simulink model "ofdm_txrx_supermimo_coded"  (Simulink 7.7)
settings: Solver=VariableStepDiscrete  SolverMode=SingleTasking  StartTime=0.0  StopTime=2*simOnly_simLength  RelTol=1e-3
PostLoadFcn:
  | ofdm_rx_supermimo_init
InitFcn:
  | ofdm_rx_supermimo_init
StartFcn:
  | ofdm_rx_supermimo_init
values from neighbouring files (symbols this model uses):
  ActionID_AFTransmit = 31    [ofdm_tx_supermimo_init.m]
  ActionID_Disabled = 0    [ofdm_tx_supermimo_init.m]
  ActionID_SetFlagA = 62    [ofdm_tx_supermimo_init.m]
  ActionID_SetFlagB = 61    [ofdm_tx_supermimo_init.m]
  BER_RAM_init_size = 2048    [ofdm_tx_supermimo_init.m]
  channel_h = [1 0; 1 0] * 0.3    [ofdm_tx_supermimo_init.m]
  CPLength = 16    [ofdm_tx_supermimo_init.m]
  longCorr_coef_i = [-4*real(LTS) 0]    [ofdm_rx_supermimo_init.m]
  longCorr_coef_q = [4*imag(LTS) 0]    [ofdm_rx_supermimo_init.m]
  max_CP_length = 16    [ofdm_tx_supermimo_init.m]
  max_num_baseRateSymbols = 31    [ofdm_tx_supermimo_init.m]
  max_num_subcarriers = 64    [ofdm_tx_supermimo_init.m]
  max_num_trainingSymbols = 15    [ofdm_tx_supermimo_init.m]
  max_numBytes = 16384    [ofdm_tx_supermimo_init.m]
  max_OFDM_symbols = 1023    [ofdm_tx_supermimo_init.m]
  modConstellation_qam16 = .75*[1 3 -1 -3]./3    [ofdm_tx_supermimo_init.m]
  modConstellation_qam64 = 0.875*[3 1 5 7 -3 -1 -5 -7]./7    [ofdm_tx_supermimo_init.m]
  modConstellation_qpsk = [1 -1]./sqrt(2)    [ofdm_tx_supermimo_init.m]
  numBaseRateSymbols = numBaseRateSymbols *2    [ofdm_tx_supermimo_init.m]
  numSubcarriers = 64    [ofdm_tx_supermimo_init.m]
  numTrainingSymbols = 2    [ofdm_tx_supermimo_init.m]
  pktTiming_count_payloadStart = pktTiming_count_lsStart + pktTiming_ls_num*pktTiming_ls_length    [ofdm_rx_supermimo_init.m]
  preamble = preamble_scale*[shortsyms_10 longsyms_2]    [ofdm_tx_supermimo_init.m]
  preamble_ROM = preamble_I_ROM + preamble_Q_ROM*2^16    [ofdm_tx_supermimo_init.m]
  RAM_init_size = max_numBytes    (= 16384)    [ofdm_tx_supermimo_init.m]
  RxDataScrambling_Seq = [40 198 78 63 82 173 102 245 48 111 172 115 147 230 216 93 72 65 62 2 205 242 122 90 128 83 105 97 73 10 5 252]    [ofdm_tx_supermimo_init.m]
  shortSymbol_time = shortSymbol_time(1:16).'    [ofdm_tx_supermimo_init.m]
  simOnly_numSamples = length(preamble)+( (numSubcarriers+CPLength)*(numTrainingSymbols + numBaseRateSymbols + numFullRateSymbols/2) )    [ofdm_tx_supermimo_init.m]
  train = [train train]    [ofdm_tx_supermimo_init.m]
  TxDataScrambling_Seq = [40 198 78 63 82 173 102 245 48 111 172 115 147 230 216 93 72 65 62 2 205 242 122 90 128 83 105 97 73 10 5 252]    [ofdm_tx_supermimo_init.m]
undefined symbols (not defined in the file or in its folder): AntA, AntB, BaseRate, Down, gcbh, numBuffs, numBytes, Up, xlBlockLoadCallback, xlGetNormalizedPeriod, xlipgetpartsetting
libraries: xbsIndex_r4 x5430
(part 30 of 49)

== system ofdm_txrx_supermimo_coded/OFDM Rx MIMO/FFT & Chan Est/Channel Estimation & Pilot Processing/H_B-B Estimate Buffer/Q Estimate Buffer ==
  note: data
  Est_Data : Inport
  Est_Addr : Inport  Port=2
  Est_WE : Inport  Port=3
  Rst : Inport  Port=4
  AddSub2 : xbsIndex_r4/AddSub  mode=Addition  use_carryin=off  use_carryout=off  en=off  latency=0  precision=User Defined  arith_type="Signed  (2's comp)"  n_bits=18  bin_pt=15  quantization=Truncate  overflow=Flag as error  dbl_ovrd=off  use_behavioral_HDL=on  hw_selection=Fabric  pipelined=off  use_rpm=on  block_type=addsub
      settings: xl_area=[0, 0, 0, 0, 0, 0, 0]  block_version=VER_STRING_GOES_HERE
  Constant Read State : xbsIndex_r4/Constant  const=0  gui_display_data_type=Fixed-point  arith_type=Boolean  n_bits=1  bin_pt=0  preci_type=Single  exp_width=8  frac_width=24  explicit_period=off  period=1  equ=P=C  opselect=C  inp2=PCIN>>17  opr=+  inp1=P  carry=CIN  dbl_ovrd=off  block_type=constant
      settings: block_version=VER_STRING_GOES_HERE
  Constant4 : xbsIndex_r4/Constant  const=0  gui_display_data_type=Fixed-point  arith_type=Signed (2's comp)  n_bits=18  bin_pt=15  preci_type=Single  exp_width=8  frac_width=24  explicit_period=off  period=1  equ=P=C  opselect=C  inp2=PCIN>>17  opr=+  inp1=P  carry=CIN  dbl_ovrd=off  block_type=constant
      settings: block_version=VER_STRING_GOES_HERE
  Convert1 : xbsIndex_r4/Convert  gui_display_data_type=Fixed-point  arith_type="Signed  (2's comp)"  n_bits=18  bin_pt=15  float_type=Single  exp_bits=8  fraction_bits=24  quantization=Truncate  overflow=Flag as error  en=off  latency=0  dbl_ovrd=off  pipeline=off  block_type=convert
      settings: xl_area=[0, 0, 0, 0, 0, 0, 0]  block_version=VER_STRING_GOES_HERE
  Convert2 : xbsIndex_r4/Convert  gui_display_data_type=Fixed-point  arith_type="Signed  (2's comp)"  n_bits=18  bin_pt=16  float_type=Single  exp_bits=8  fraction_bits=24  quantization=Truncate  overflow=Flag as error  en=off  latency=0  dbl_ovrd=off  pipeline=off  block_type=convert
      settings: xl_area=[0, 0, 0, 0, 0, 0, 0]  block_version=VER_STRING_GOES_HERE
  Delay3 : xbsIndex_r4/Delay  rst=off  infoeditControl=Selection of Reset will increase slice count due to use of real FFs and instead of SRLs  en=off  latency=1  dbl_ovrd=off  reg_retiming=off  block_type=delay
      settings: infoedit=Hardware notes: A delay line is a chain, each link of which is an SRL16 followed by a flip-flop. If register retiming is enabled, the delay line is a chain of flip-flops.  xl_area=[0, 0, 0, 0, 0, 0, 0]  block_version=VER_STRING_GOES_HERE
  Delay6 : xbsIndex_r4/Delay  rst=off  infoeditControl=Selection of Reset will increase slice count due to use of real FFs and instead of SRLs  en=off  latency=1  dbl_ovrd=off  reg_retiming=off  block_type=delay
      settings: infoedit=Hardware notes: A delay line is a chain, each link of which is an SRL16 followed by a flip-flop. If register retiming is enabled, the delay line is a chain of flip-flops.  xl_area=[0, 0, 0, 0, 0, 0, 0]  block_version=VER_STRING_GOES_HERE
  Delay7 : xbsIndex_r4/Delay  rst=off  infoeditControl=Selection of Reset will increase slice count due to use of real FFs and instead of SRLs  en=off  latency=1  dbl_ovrd=off  reg_retiming=off  block_type=delay
      settings: infoedit=Hardware notes: A delay line is a chain, each link of which is an SRL16 followed by a flip-flop. If register retiming is enabled, the delay line is a chain of flip-flops.  xl_area=[0, 0, 0, 0, 0, 0, 0]  block_version=VER_STRING_GOES_HERE
  Dual Port RAM : xbsIndex_r4/Dual Port RAM  depth=numSubcarriers  initVector=zeros(1,numSubcarriers)  distributed_mem=Block RAM  init_a=0  init_b=0  rst_a=off  rst_b=on  en_a=off  en_b=off  latency=1  write_mode_A=Read Before Write  write_mode_B=Read After Write  dbl_ovrd=off  optimize=Area  use_rpm=off  block_type=dpram
      settings: xl_area=[0, 0, 0, 0, 0, 0, 0]  block_version=VER_STRING_GOES_HERE
  h[n] : Outport
  wires:
  Constant4/1 -> Dual Port RAM/5
  Constant Read State/1 -> Dual Port RAM/6
  AddSub2/1 -> Dual Port RAM/2
  Convert1/1 -> AddSub2/1
  Delay6/1 -> Dual Port RAM/1
  Delay3/1 -> Dual Port RAM/3
  Delay7/1 -> AddSub2/2
  Est_WE/1 -> Delay3/1
  Est_Addr/1 -> Dual Port RAM/4, Delay6/1
  Rst/1 -> Dual Port RAM/7
  Est_Data/1 -> Delay7/1
  Dual Port RAM/2 -> Convert2/1, Convert1/1
  Convert2/1 -> h[n]/1

== system ofdm_txrx_supermimo_coded/OFDM Rx MIMO/FFT & Chan Est/Channel Estimation & Pilot Processing/Pilot Processing/CFO Calculation ==
  Phase Err : Inport
  Vin : Inport  Port=2
  Rst : Inport  Port=3
  Add : Sum  Inputs=+-
  Constant : Constant
  Correction : Subsystem
  Delay6 : xbsIndex_r4/Delay  rst=off  infoeditControl=Selection of Reset will increase slice count due to use of real FFs and instead of SRLs  en=on  latency=1  dbl_ovrd=off  reg_retiming=off  block_type=delay
      settings: infoedit=Hardware notes: A delay line is a chain, each link of which is an SRL16 followed by a flip-flop. If register retiming is enabled, the delay line is a chain of flip-flops.  block_version=VER_STRING_GOES_HERE
  Diff Calc : Subsystem
  Gateway Out{1..4,8} (5 alike) : xbsIndex_r4/Gateway Out  inherit_from_input=off  hdl_port=off  timing_constraint=None  locs_specified=off  LOCs={}  UseAsDAC=off  DACChannel='1'  block_type=gatewayout
      settings: infoedit="Gateway out block.  Converts Xilinx fixed point inputs into ouputs of type Simulink integer, double, or fixed point.<P><P>Hardware notes:  In hardware these blocks become top level output ports or are discarded, depending on how they are configured."  block_version=VER_STRING_GOES_HERE
  Unwrapper : Subsystem
  CFO : Outport
  Vout : Outport  Port=2
  wires:
  Phase Err/1 -> Unwrapper/1(Phase), Gateway Out4/1
  Vin/1 -> Unwrapper/2(Vin), Gateway Out2/1
  Gateway Out8/1 -> Add/2
  Gateway Out2/1 -> ?
  Gateway Out4/1 -> ?
  Rst/1 -> Unwrapper/3(Rst), Diff Calc/3(Rst)
  Add/1 -> ?
  Constant/1 -> Add/1
  Unwrapper/1(" Phase") -> Diff Calc/1(Phase Err)
  Unwrapper/2(Vout) -> Diff Calc/2(Vin)
  Diff Calc/2(Vout) -> Correction/2(Vin)
  Diff Calc/1(AvgErr) -> Correction/1(Phase Err)
  Correction/1(AvgErr) -> CFO/1, Delay6/1, Gateway Out8/1
  Correction/2(Vout) -> Vout/1, Delay6/2, Gateway Out1/1
  Gateway Out1/1 -> ?
  Gateway Out3/1 -> ?
  Delay6/1 -> Gateway Out3/1

== system ofdm_txrx_supermimo_coded/OFDM Rx MIMO/FFT & Chan Est/Channel Estimation & Pilot Processing/Pilot Processing/Phase Noise Tracker ==
  note: http://ieeexplore.ieee.org/xpls/abs_all.jsp?arnumber=1683187
  note: Adapted from the phase tracking system described by Akita, Sakata and Sato
  note: http://sciencelinks.jp/j-east/article/200415/000020041504A0494348.php
  note: http://ieeexplore.ieee.org/xpls/abs_all.jsp?arnumber=1405003
  Phase Err : Inport
  Reset : Inport  Port=2
  Vin : Inport  Port=3
  Sym Index : Inport  Port=4
  Bus Creator1 : BusCreator  Inputs=2
  Bus Creator2 : BusCreator  Inputs=2
  Coarse Tracking : Subsystem
  Convert1 : xbsIndex_r4/Convert  gui_display_data_type=Fixed-point  arith_type="Signed  (2's comp)"  n_bits=14  bin_pt=13  float_type=Single  exp_bits=8  fraction_bits=24  quantization=Truncate  overflow=Wrap  en=off  latency=0  dbl_ovrd=off  pipeline=off  block_type=convert
      settings: xl_area=[0, 0, 0, 0, 0, 0, 0]  block_version=VER_STRING_GOES_HERE
  Convert2 : xbsIndex_r4/Convert  gui_display_data_type=Fixed-point  arith_type="Signed  (2's comp)"  n_bits=16  bin_pt=16  float_type=Single  exp_bits=8  fraction_bits=24  quantization=Truncate  overflow=Wrap  en=off  latency=0  dbl_ovrd=off  pipeline=off  block_type=convert
      settings: xl_area=[0, 0, 0, 0, 0, 0, 0]  block_version=VER_STRING_GOES_HERE
  "DDS Compiler 4.0 " : xbsIndex_r4/DDS Compiler 4.0   partspresent=SIN_COS_LUT_only  dds_clock_rate=100  channels=1  parameter_entry=Hardware_Parameters  spurious_free_dynamic_range=36  frequency_resolution=0.4  noise_shaping=None  phase_width=16  output_width=16  output_selection=Sine_and_Cosine  negative_sine=on  negative_cosine=off  amplitude_mode=Full_Range  memory_type=Auto  optimization_goal=Auto  dsp48_use=Minimal  latency_configuration=Auto  latency=6  has_phase_out=off  sclr_pin=off  clock_enable=on  rfd=off  rdy=off  channel_pin=off  explicit_period=off  period=1  phase_increment=Fixed  output_frequency1=0  output_frequency2=0  output_frequency3=0  output_frequency4=0  output_frequency5=0  output_frequency6=0  output_frequency7=0  output_frequency8=0  output_frequency9=0  output_frequency10=0  output_frequency11=0  output_frequency12=0  output_frequency13=0  output_frequency14=0  output_frequency15=0  output_frequency16=0  pinc1='0'  pinc2='0'  pinc3='0'  pinc4='0'  pinc5='0'  pinc6='0'  pinc7='0'  pinc8='0'  pinc9='0'  pinc10='0'  pinc11='0'  pinc12='0'  pinc13='0'  pinc14='0'  pinc15='0'  pinc16='0'  phase_offset=None  phase_offset_angles1=0  phase_offset_angles2=0  phase_offset_angles3=0  phase_offset_angles4=0  phase_offset_angles5=0  phase_offset_angles6=0  phase_offset_angles7=0  phase_offset_angles8=0  phase_offset_angles9=0  phase_offset_angles10=0  phase_offset_angles11=0  phase_offset_angles12=0  phase_offset_angles13=0  phase_offset_angles14=0  phase_offset_angles15=0  phase_offset_angles16=0  poff1='0'  poff2='0'  poff3='0'  poff4='0'  poff5='0'  poff6='0'  poff7='0'  poff8='0'  poff9='0'  poff10='0'  poff11='0'  poff12='0'  poff13='0'  poff14='0'  poff15='0'  poff16='0'  por_mode=false  gui_behaviour=Sysgen  ip_name=DDS Compiler  ip_version=4.0  dsptool_ready=true  wrapper_available=true  port_translation_map={ 'ce' => 'en', 'sclr' => 'rst' }  ipcore_xco_need_fpga_part=true  ipcore_fpga_part=xlipgetpartsetting(gcb, {'virtex4', 'xc4vsx35', '-10', 'ff668'})  ipcore_usecache=true  ipcore_useipmodelcache=true  ipcore_verbose=false  block_type=dds_compiler_v4_0
  Delay1 : xbsIndex_r4/Delay  rst=off  infoeditControl=Selection of Reset will increase slice count due to use of real FFs and instead of SRLs  en=off  latency=1  dbl_ovrd=off  reg_retiming=off  block_type=delay
      settings: infoedit=Hardware notes: A delay line is a chain, each link of which is an SRL16 followed by a flip-flop. If register retiming is enabled, the delay line is a chain of flip-flops.  block_version=VER_STRING_GOES_HERE
  Delay3 : xbsIndex_r4/Delay  rst=off  infoeditControl=Selection of Reset will increase slice count due to use of real FFs and instead of SRLs  en=off  latency=1  dbl_ovrd=off  reg_retiming=off  block_type=delay
      settings: infoedit=Hardware notes: A delay line is a chain, each link of which is an SRL16 followed by a flip-flop. If register retiming is enabled, the delay line is a chain of flip-flops.  block_version=VER_STRING_GOES_HERE
  Down Sample{1..4} (4 alike) : xbsIndex_r4/Down Sample  sample_ratio=2  sample_phase="Last Value of Frame  (most efficient)"  en=off  latency=1  dbl_ovrd=off  block_type=dsamp
      settings: block_version=10.1.3
  Fine Tracking : Subsystem
  Gateway Out{,1..5} (6 alike) : xbsIndex_r4/Gateway Out  inherit_from_input=off  hdl_port=off  timing_constraint=None  locs_specified=off  LOCs={}  UseAsDAC=off  DACChannel='1'  block_type=gatewayout
      settings: infoedit="Gateway out block.  Converts Xilinx fixed point inputs into ouputs of type Simulink integer, double, or fixed point.<P><P>Hardware notes:  In hardware these blocks become top level output ports or are discarded, depending on how they are configured."  block_version=10.1.3
  Logical : xbsIndex_r4/Logical  logical_function=OR  inputs=2  en=off  latency=0  precision=Full  arith_type=Unsigned  n_bits=16  bin_pt=0  align_bp=on  dbl_ovrd=off  block_type=logical
  Register{2..5} (4 alike) : xbsIndex_r4/Register  init=0  rst=off  en=on  dbl_ovrd=off  block_type=register
  Rx_debug_PhaseCorrect : xbsIndex_r4/Gateway Out  inherit_from_input=off  hdl_port=on  timing_constraint=None  locs_specified=off  LOCs={}  UseAsDAC=off  DACChannel='1'  block_type=gatewayout
      settings: infoedit="Gateway out block.  Converts Xilinx fixed point inputs into ouputs of type Simulink integer, double, or fixed point.<P><P>Hardware notes:  In hardware these blocks become top level output ports or are discarded, depending on how they are configured."  xl_area=[0 0 0 0 1 0 0]  block_version=VER_STRING_GOES_HERE
  Phases_I : Outport
  Phases_Q : Outport  Port=2
  wires:
  Phase Err/1 -> Coarse Tracking/1(Phase Err)
  Vin/1 -> Coarse Tracking/2(Vin)
  Coarse Tracking/1(phi) -> Fine Tracking/1(phi)
  Coarse Tracking/2(theta_coarse) -> Fine Tracking/2(theta_coarse)
  Sym Index/1 -> Fine Tracking/5(Sym Index)
  Coarse Tracking/3(vout) -> Fine Tracking/3(Vin)
  Fine Tracking/1(theta) -> Convert2/1
  Reset/1 -> Coarse Tracking/3(Rst), Fine Tracking/4(Rst), Gateway Out/1
  "DDS Compiler 4.0 "/1 -> Register2/1, Register3/1, Gateway Out4/1
  "DDS Compiler 4.0 "/2 -> Register4/1, Register5/1, Gateway Out3/1
  Convert1/1 -> Rx_debug_PhaseCorrect/1
  Fine Tracking/2(r0_vout) -> Logical/1, Delay3/1, Gateway Out1/1
  Convert2/1 -> Convert1/1, "DDS Compiler 4.0 "/1
  Down Sample4/1 -> Bus Creator1/1
  Bus Creator1/1 -> Phases_Q/1
  Bus Creator2/1 -> Phases_I/1
  Down Sample3/1 -> Bus Creator1/2
  Down Sample2/1 -> Bus Creator2/1
  Down Sample1/1 -> Bus Creator2/2
  Fine Tracking/3(r1_vout) -> Logical/2, Delay1/1, Gateway Out2/1
  Delay3/1 -> Register2/2, Register4/2
  Delay1/1 -> Register3/2, Register5/2
  Logical/1 -> "DDS Compiler 4.0 "/2
  Register2/1 -> Down Sample4/1, Gateway Out5/1
  Register3/1 -> Down Sample3/1
  Register4/1 -> Down Sample2/1
  Register5/1 -> Down Sample1/1
  Gateway Out/1 -> ?
  Gateway Out1/1 -> ?
  Gateway Out2/1 -> ?
  Gateway Out3/1 -> ?
  Gateway Out4/1 -> ?
  Gateway Out5/1 -> ?

== system ofdm_txrx_supermimo_coded/OFDM Rx MIMO/FFT & Chan Est/Channel Estimation & Pilot Processing/Pilot Processing/Pilot Phase Error Calc ==
  Raw I : Inport
  Raw Q : Inport  Port=2
  Xk_index : Inport  Port=3
  Sym Index : Inport  Port=4
  H_AA I : Inport  Port=5
  H_AA Q : Inport  Port=6
  H_BA I : Inport  Port=7
  H_BA Q : Inport  Port=8
  Train : Inport  Port=9
  "Rst " : Inport  Port=10
  Averager : Subsystem
  Chan Phase RAM : Subsystem
  Gateway Out{1..3,7..10} (7 alike) : xbsIndex_r4/Gateway Out  inherit_from_input=off  hdl_port=off  timing_constraint=None  locs_specified=off  LOCs={}  UseAsDAC=off  DACChannel='1'  block_type=gatewayout
      settings: infoedit="Gateway out block.  Converts Xilinx fixed point inputs into ouputs of type Simulink integer, double, or fixed point.<P><P>Hardware notes:  In hardware these blocks become top level output ports or are discarded, depending on how they are configured."  block_version=VER_STRING_GOES_HERE
  Phase Calculation : Subsystem
  Pilot_Descrambler : Subsystem
  S-R Latch : Subsystem
  negedge : Subsystem
  posedge1 : Subsystem
  Phase Err : Outport
  Rst : Outport  Port=2
  Vout : Outport  Port=3
  " Sym Index" : Outport  Port=4
  wires:
  S-R Latch/1(D) -> Rst/1
  Xk_index/1 -> Pilot_Descrambler/2(FFT Index), Phase Calculation/7(Subcar Ind)
  Raw I/1 -> Pilot_Descrambler/4("I ")
  Raw Q/1 -> Pilot_Descrambler/5("Q ")
  Sym Index/1 -> Pilot_Descrambler/1(Sym Index), Phase Calculation/6(Sym Index)
  Pilot_Descrambler/1(I) -> Phase Calculation/8(Pilot I)
  Pilot_Descrambler/2(Q) -> Phase Calculation/9(Pilot Q)
  H_AA I/1 -> Phase Calculation/2(H_AA I)
  H_AA Q/1 -> Phase Calculation/3(H_AA Q)
  H_BA I/1 -> Phase Calculation/4(H_BA I)
  H_BA Q/1 -> Phase Calculation/5(H_BA Q)
  Phase Calculation/2(Phase) -> Chan Phase RAM/3(Phase), Gateway Out7/1
  Chan Phase RAM/1(d(Phase)) -> Averager/2(d(Phase))
  Train/1 -> Phase Calculation/1(Train), posedge1/1(D), negedge/1(D)
  negedge/1(Q) -> S-R Latch/1(R)
  Averager/1(Phase Err) -> Phase Err/1
  Averager/2(Vout) -> Vout/1
  Phase Calculation/1(" Train") -> Chan Phase RAM/2(Train), Gateway Out8/1
  Pilot_Descrambler/3(A_Pilot) -> Phase Calculation/10("A_Pilot ")
  Pilot_Descrambler/4(B_Pilot) -> Phase Calculation/11("B_Pilot ")
  Phase Calculation/3(H_xA Vout) -> Gateway Out9/1, Chan Phase RAM/4(H_xA Vin)
  Phase Calculation/4(A_Pilot Vout) -> Gateway Out10/1, Chan Phase RAM/5(A Pilot Vin)
  Phase Calculation/5(B_Pilot Vout) -> Gateway Out1/1, Chan Phase RAM/6(B Pilot Vin)
  Phase Calculation/6(Use A Pilots) -> Chan Phase RAM/7(Use A Pilots), Gateway Out2/1
  Phase Calculation/7(Use B Pilots) -> Chan Phase RAM/8(Use B Pilots), Gateway Out3/1
  Chan Phase RAM/2(Vout) -> Averager/3(Vin)
  Chan Phase RAM/3(Use Both) -> Averager/4(Use Both)
  Gateway Out8/1 -> ?
  Gateway Out7/1 -> ?
  Gateway Out9/1 -> ?
  Gateway Out10/1 -> ?
  Gateway Out1/1 -> ?
  Gateway Out2/1 -> ?
  Gateway Out3/1 -> ?
  Phase Calculation/8(" Sym Index") -> Chan Phase RAM/9(Sym Index)
  Chan Phase RAM/4(" Sym Index") -> Averager/5(Sym Index)
  Averager/3(" Sym Index") -> " Sym Index"/1
  "Rst "/1 -> Pilot_Descrambler/3(rst), Chan Phase RAM/1(Rst), Averager/1(Rst), S-R Latch/2(S)

== system ofdm_txrx_supermimo_coded/OFDM Rx MIMO/FFT & Chan Est/FFT/FreqSelective "Fading"/FreqSelective "Fading" ==
  note: Scales one subcarrier value by a constant, simulating
      | a deep frequency selective fade. Used to gauge impact
      | of fixed point precision issues in equalizer. Ignored
      | when generating model for hardware.
  I : Inport
  Q : Inport  Port=2
  Ind : Inport  Port=3
  Constant : Constant  Value=10
  Disregard Subsystem : xbsIndex_r4/Disregard Subsystem  Tag=discardX  block_type=disregard
      settings: block_version=10.1.3
  Gain1 : Gain
  Gain2 : Gain
  Gateway In : xbsIndex_r4/Gateway In  gui_display_data_type=Fixed-point  arith_type="Signed  (2's comp)"  n_bits=16  bin_pt=15  preci_type=Single  exp_width=8  frac_width=24  quantization="Round  (unbiased: +/- Inf)"  overflow=Saturate  period=1  dbl_ovrd=off  timing_constraint=None  locs_specified=off  LOCs={}  inherit_from_input=off  UseAsADC=off  ADCChannel='1'  hdl_port=on  block_type=gatewayin
      settings: infoedit="Gateway in block.  Converts inputs of type Simulink integer, double and fixed point to  Xilinx fixed point type.<P><P>Hardware notes:  In hardware these blocks become top level input ports."  block_version=10.1.3
  Gateway In1 : xbsIndex_r4/Gateway In  gui_display_data_type=Fixed-point  arith_type="Signed  (2's comp)"  n_bits=16  bin_pt=15  preci_type=Single  exp_width=8  frac_width=24  quantization="Round  (unbiased: +/- Inf)"  overflow=Saturate  period=1  dbl_ovrd=off  timing_constraint=None  locs_specified=off  LOCs={}  inherit_from_input=off  UseAsADC=off  ADCChannel='1'  hdl_port=on  block_type=gatewayin
      settings: infoedit="Gateway in block.  Converts inputs of type Simulink integer, double and fixed point to  Xilinx fixed point type.<P><P>Hardware notes:  In hardware these blocks become top level input ports."  block_version=10.1.3
  Gateway Out : xbsIndex_r4/Gateway Out  inherit_from_input=off  hdl_port=off  timing_constraint=None  locs_specified=off  LOCs={}  UseAsDAC=off  DACChannel='1'  block_type=gatewayout
      settings: infoedit="Gateway out block.  Converts Xilinx fixed-point or floating-point type inputs into ouputs of type Simulink integer, single, double, or fixed point.<br><br>Hardware notes:  In hardware these blocks become top level output ports or are discarded, depending on how they are configured."  block_version=10.1.3
  Gateway Out1 : xbsIndex_r4/Gateway Out  inherit_from_input=off  hdl_port=off  timing_constraint=None  locs_specified=off  LOCs={}  UseAsDAC=off  DACChannel='1'  block_type=gatewayout
      settings: infoedit="Gateway out block.  Converts Xilinx fixed-point or floating-point type inputs into ouputs of type Simulink integer, single, double, or fixed point.<br><br>Hardware notes:  In hardware these blocks become top level output ports or are discarded, depending on how they are configured."  block_version=10.1.3
  Relational Operator : RelationalOperator  Operator===  OutDataTypeStr=boolean
  Switch : Switch
  Switch1 : Switch  Criteria=u2 ~= 0
  done7 : xbsIndex_r4/Gateway Out  inherit_from_input=off  hdl_port=off  timing_constraint=None  locs_specified=off  LOCs={}  UseAsDAC=off  DACChannel='1'  block_type=gatewayout
      settings: infoedit="Gateway out block.  Converts Xilinx fixed point inputs into ouputs of type Simulink integer, double, or fixed point.<P><P>Hardware notes:  In hardware these blocks become top level output ports or are discarded, depending on how they are configured."  xl_area=[0 0 0 0 1 0 0]  block_version=VER_STRING_GOES_HERE
  " I" : Outport
  " Q" : Outport  Port=2
  wires:
  Gain1/1 -> Switch1/1
  Switch/1 -> Gateway In/1
  Switch1/1 -> Gateway In1/1
  Gateway In/1 -> " I"/1
  Gateway In1/1 -> " Q"/1
  Gain2/1 -> Switch/1
  Gateway Out/1 -> Gain2/1, Switch/3
  Relational Operator/1 -> Switch1/2, Switch/2
  Constant/1 -> Relational Operator/1
  Ind/1 -> done7/1
  I/1 -> Gateway Out/1
  Q/1 -> Gateway Out1/1
  Gateway Out1/1 -> Gain1/1, Switch1/3
  done7/1 -> Relational Operator/2

== system ofdm_txrx_supermimo_coded/OFDM Rx MIMO/FFT & Chan Est/FFT/Ind Chk1/Indeterminate Check ==
  In1 : Inport
  Vin : Inport  Port=2
  Constant : xbsIndex_r4/Constant  const=0  gui_display_data_type=Fixed-point  arith_type=Signed (2's comp)  n_bits=16  bin_pt=15  preci_type=Single  exp_width=8  frac_width=24  explicit_period=off  period=1  equ=P=C  opselect=C  inp2=PCIN>>17  opr=+  inp1=P  carry=CIN  dbl_ovrd=off  block_type=constant
  Disregard Subsystem : xbsIndex_r4/Disregard Subsystem  Tag=discardX  block_type=disregard
      settings: block_version=10.1.3
  Mux1 : xbsIndex_r4/Mux  inputs=2  en=off  latency=0  precision=Full  arith_type=Unsigned  n_bits=16  bin_pt=14  quantization=Truncate  overflow=Wrap  dbl_ovrd=off  block_type=mux
  Out1 : Outport
  wires:
  In1/1 -> Mux1/3
  Vin/1 -> Mux1/1
  Constant/1 -> Mux1/2
  Mux1/1 -> Out1/1

== system ofdm_txrx_supermimo_coded/OFDM Rx MIMO/FFT & Chan Est/FFT/Ind Chk2/Indeterminate Check ==
  In1 : Inport
  Vin : Inport  Port=2
  Constant : xbsIndex_r4/Constant  const=0  gui_display_data_type=Fixed-point  arith_type=Signed (2's comp)  n_bits=16  bin_pt=15  preci_type=Single  exp_width=8  frac_width=24  explicit_period=off  period=1  equ=P=C  opselect=C  inp2=PCIN>>17  opr=+  inp1=P  carry=CIN  dbl_ovrd=off  block_type=constant
  Disregard Subsystem : xbsIndex_r4/Disregard Subsystem  Tag=discardX  block_type=disregard
      settings: block_version=10.1.3
  Mux1 : xbsIndex_r4/Mux  inputs=2  en=off  latency=0  precision=Full  arith_type=Unsigned  n_bits=16  bin_pt=14  quantization=Truncate  overflow=Wrap  dbl_ovrd=off  block_type=mux
  Out1 : Outport
  wires:
  Mux1/1 -> Out1/1
  Constant/1 -> Mux1/2
  Vin/1 -> Mux1/1
  In1/1 -> Mux1/3

== system ofdm_txrx_supermimo_coded/OFDM Rx MIMO/Long Corr HP/C1/C1/MAC ==
  a : Inport
  coeff : Inport  Port=2
  ld : Inport  Port=3
  AddSub : xbsIndex_r4/AddSub  mode=Addition or Subtraction  use_carryin=off  use_carryout=off  en=off  latency=0  precision=User Defined  arith_type="Signed  (2's comp)"  n_bits=long_cor_acc_n_bits  bin_pt=0  quantization=Truncate  overflow=Wrap  dbl_ovrd=off  use_behavioral_HDL=on  hw_selection=Fabric  pipelined=off  use_rpm=off  block_type=addsub
      settings: xl_area=[2 0 0 4 0 0 0]  block_version=VER_STRING_GOES_HERE
  Register1 : xbsIndex_r4/Register  init=0  rst=on  en=off  dbl_ovrd=off  block_type=register
      settings: xl_area=[2 4 0 0 0 0 0]  block_version=VER_STRING_GOES_HERE
  Register2 : xbsIndex_r4/Register  init=0  rst=off  en=on  dbl_ovrd=off  block_type=register
      settings: xl_area=[2 4 0 0 0 0 0]  block_version=VER_STRING_GOES_HERE
  bc2 : xbsIndex_r4/Convert  gui_display_data_type=Fixed-point  arith_type=Boolean  n_bits=1  bin_pt=0  float_type=Single  exp_bits=8  fraction_bits=24  quantization=Truncate  overflow=Wrap  en=off  latency=0  dbl_ovrd=off  pipeline=off  block_type=convert
      settings: xl_area=[0 0 0 0 0 0 0]  block_version=VER_STRING_GOES_HERE
  bc3 : xbsIndex_r4/Convert  gui_display_data_type=Fixed-point  arith_type=Boolean  n_bits=1  bin_pt=0  float_type=Single  exp_bits=8  fraction_bits=24  quantization=Truncate  overflow=Wrap  en=off  latency=0  dbl_ovrd=off  pipeline=off  block_type=convert
      settings: xl_area=[0 0 0 0 0 0 0]  block_version=VER_STRING_GOES_HERE
  yn : Outport
  wires:
  a/1 -> bc2/1
  AddSub/1 -> Register1/1, Register2/1
  Register2/1 -> yn/1
  Register1/1 -> AddSub/1
  ld/1 -> bc3/1
  bc3/1 -> Register2/2, Register1/2
  bc2/1 -> AddSub/3
  coeff/1 -> AddSub/2

== system ofdm_txrx_supermimo_coded/OFDM Rx MIMO/Long Corr HP/C1/C10/MAC ==
  a : Inport
  coeff : Inport  Port=2
  ld : Inport  Port=3
  AddSub : xbsIndex_r4/AddSub  mode=Addition or Subtraction  use_carryin=off  use_carryout=off  en=off  latency=0  precision=User Defined  arith_type="Signed  (2's comp)"  n_bits=long_cor_acc_n_bits  bin_pt=0  quantization=Truncate  overflow=Wrap  dbl_ovrd=off  use_behavioral_HDL=on  hw_selection=Fabric  pipelined=off  use_rpm=off  block_type=addsub
      settings: xl_area=[2 0 0 4 0 0 0]  block_version=VER_STRING_GOES_HERE
  Register1 : xbsIndex_r4/Register  init=0  rst=on  en=off  dbl_ovrd=off  block_type=register
      settings: xl_area=[2 4 0 0 0 0 0]  block_version=VER_STRING_GOES_HERE
  Register2 : xbsIndex_r4/Register  init=0  rst=off  en=on  dbl_ovrd=off  block_type=register
      settings: xl_area=[2 4 0 0 0 0 0]  block_version=VER_STRING_GOES_HERE
  bc2 : xbsIndex_r4/Convert  gui_display_data_type=Fixed-point  arith_type=Boolean  n_bits=1  bin_pt=0  float_type=Single  exp_bits=8  fraction_bits=24  quantization=Truncate  overflow=Wrap  en=off  latency=0  dbl_ovrd=off  pipeline=off  block_type=convert
      settings: xl_area=[0 0 0 0 0 0 0]  block_version=VER_STRING_GOES_HERE
  bc3 : xbsIndex_r4/Convert  gui_display_data_type=Fixed-point  arith_type=Boolean  n_bits=1  bin_pt=0  float_type=Single  exp_bits=8  fraction_bits=24  quantization=Truncate  overflow=Wrap  en=off  latency=0  dbl_ovrd=off  pipeline=off  block_type=convert
      settings: xl_area=[0 0 0 0 0 0 0]  block_version=VER_STRING_GOES_HERE
  yn : Outport
  wires:
  a/1 -> bc2/1
  AddSub/1 -> Register1/1, Register2/1
  Register2/1 -> yn/1
  Register1/1 -> AddSub/1
  ld/1 -> bc3/1
  bc3/1 -> Register2/2, Register1/2
  bc2/1 -> AddSub/3
  coeff/1 -> AddSub/2

== system ofdm_txrx_supermimo_coded/OFDM Rx MIMO/Long Corr HP/C1/C11/MAC ==
  a : Inport
  coeff : Inport  Port=2
  ld : Inport  Port=3
  AddSub : xbsIndex_r4/AddSub  mode=Addition or Subtraction  use_carryin=off  use_carryout=off  en=off  latency=0  precision=User Defined  arith_type="Signed  (2's comp)"  n_bits=long_cor_acc_n_bits  bin_pt=0  quantization=Truncate  overflow=Wrap  dbl_ovrd=off  use_behavioral_HDL=on  hw_selection=Fabric  pipelined=off  use_rpm=off  block_type=addsub
      settings: xl_area=[2 0 0 4 0 0 0]  block_version=VER_STRING_GOES_HERE
  Register1 : xbsIndex_r4/Register  init=0  rst=on  en=off  dbl_ovrd=off  block_type=register
      settings: xl_area=[2 4 0 0 0 0 0]  block_version=VER_STRING_GOES_HERE
  Register2 : xbsIndex_r4/Register  init=0  rst=off  en=on  dbl_ovrd=off  block_type=register
      settings: xl_area=[2 4 0 0 0 0 0]  block_version=VER_STRING_GOES_HERE
  bc2 : xbsIndex_r4/Convert  gui_display_data_type=Fixed-point  arith_type=Boolean  n_bits=1  bin_pt=0  float_type=Single  exp_bits=8  fraction_bits=24  quantization=Truncate  overflow=Wrap  en=off  latency=0  dbl_ovrd=off  pipeline=off  block_type=convert
      settings: xl_area=[0 0 0 0 0 0 0]  block_version=VER_STRING_GOES_HERE
  bc3 : xbsIndex_r4/Convert  gui_display_data_type=Fixed-point  arith_type=Boolean  n_bits=1  bin_pt=0  float_type=Single  exp_bits=8  fraction_bits=24  quantization=Truncate  overflow=Wrap  en=off  latency=0  dbl_ovrd=off  pipeline=off  block_type=convert
      settings: xl_area=[0 0 0 0 0 0 0]  block_version=VER_STRING_GOES_HERE
  yn : Outport
  wires:
  a/1 -> bc2/1
  AddSub/1 -> Register1/1, Register2/1
  Register2/1 -> yn/1
  Register1/1 -> AddSub/1
  ld/1 -> bc3/1
  bc3/1 -> Register2/2, Register1/2
  bc2/1 -> AddSub/3
  coeff/1 -> AddSub/2

== system ofdm_txrx_supermimo_coded/OFDM Rx MIMO/Long Corr HP/C1/C12/MAC ==
  a : Inport
  coeff : Inport  Port=2
  ld : Inport  Port=3
  AddSub : xbsIndex_r4/AddSub  mode=Addition or Subtraction  use_carryin=off  use_carryout=off  en=off  latency=0  precision=User Defined  arith_type="Signed  (2's comp)"  n_bits=long_cor_acc_n_bits  bin_pt=0  quantization=Truncate  overflow=Wrap  dbl_ovrd=off  use_behavioral_HDL=on  hw_selection=Fabric  pipelined=off  use_rpm=off  block_type=addsub
      settings: xl_area=[2 0 0 4 0 0 0]  block_version=VER_STRING_GOES_HERE
  Register1 : xbsIndex_r4/Register  init=0  rst=on  en=off  dbl_ovrd=off  block_type=register
      settings: xl_area=[2 4 0 0 0 0 0]  block_version=VER_STRING_GOES_HERE
  Register2 : xbsIndex_r4/Register  init=0  rst=off  en=on  dbl_ovrd=off  block_type=register
      settings: xl_area=[2 4 0 0 0 0 0]  block_version=VER_STRING_GOES_HERE
  bc2 : xbsIndex_r4/Convert  gui_display_data_type=Fixed-point  arith_type=Boolean  n_bits=1  bin_pt=0  float_type=Single  exp_bits=8  fraction_bits=24  quantization=Truncate  overflow=Wrap  en=off  latency=0  dbl_ovrd=off  pipeline=off  block_type=convert
      settings: xl_area=[0 0 0 0 0 0 0]  block_version=VER_STRING_GOES_HERE
  bc3 : xbsIndex_r4/Convert  gui_display_data_type=Fixed-point  arith_type=Boolean  n_bits=1  bin_pt=0  float_type=Single  exp_bits=8  fraction_bits=24  quantization=Truncate  overflow=Wrap  en=off  latency=0  dbl_ovrd=off  pipeline=off  block_type=convert
      settings: xl_area=[0 0 0 0 0 0 0]  block_version=VER_STRING_GOES_HERE
  yn : Outport
  wires:
  a/1 -> bc2/1
  AddSub/1 -> Register1/1, Register2/1
  Register2/1 -> yn/1
  Register1/1 -> AddSub/1
  ld/1 -> bc3/1
  bc3/1 -> Register2/2, Register1/2
  bc2/1 -> AddSub/3
  coeff/1 -> AddSub/2

== system ofdm_txrx_supermimo_coded/OFDM Rx MIMO/Long Corr HP/C1/C13/MAC ==
  a : Inport
  coeff : Inport  Port=2
  ld : Inport  Port=3
  AddSub : xbsIndex_r4/AddSub  mode=Addition or Subtraction  use_carryin=off  use_carryout=off  en=off  latency=0  precision=User Defined  arith_type="Signed  (2's comp)"  n_bits=long_cor_acc_n_bits  bin_pt=0  quantization=Truncate  overflow=Wrap  dbl_ovrd=off  use_behavioral_HDL=on  hw_selection=Fabric  pipelined=off  use_rpm=off  block_type=addsub
      settings: xl_area=[2 0 0 4 0 0 0]  block_version=VER_STRING_GOES_HERE
  Register1 : xbsIndex_r4/Register  init=0  rst=on  en=off  dbl_ovrd=off  block_type=register
      settings: xl_area=[2 4 0 0 0 0 0]  block_version=VER_STRING_GOES_HERE
  Register2 : xbsIndex_r4/Register  init=0  rst=off  en=on  dbl_ovrd=off  block_type=register
      settings: xl_area=[2 4 0 0 0 0 0]  block_version=VER_STRING_GOES_HERE
  bc2 : xbsIndex_r4/Convert  gui_display_data_type=Fixed-point  arith_type=Boolean  n_bits=1  bin_pt=0  float_type=Single  exp_bits=8  fraction_bits=24  quantization=Truncate  overflow=Wrap  en=off  latency=0  dbl_ovrd=off  pipeline=off  block_type=convert
      settings: xl_area=[0 0 0 0 0 0 0]  block_version=VER_STRING_GOES_HERE
  bc3 : xbsIndex_r4/Convert  gui_display_data_type=Fixed-point  arith_type=Boolean  n_bits=1  bin_pt=0  float_type=Single  exp_bits=8  fraction_bits=24  quantization=Truncate  overflow=Wrap  en=off  latency=0  dbl_ovrd=off  pipeline=off  block_type=convert
      settings: xl_area=[0 0 0 0 0 0 0]  block_version=VER_STRING_GOES_HERE
  yn : Outport
  wires:
  a/1 -> bc2/1
  AddSub/1 -> Register1/1, Register2/1
  Register2/1 -> yn/1
  Register1/1 -> AddSub/1
  ld/1 -> bc3/1
  bc3/1 -> Register2/2, Register1/2
  bc2/1 -> AddSub/3
  coeff/1 -> AddSub/2

== system ofdm_txrx_supermimo_coded/OFDM Rx MIMO/Long Corr HP/C1/C14/MAC ==
  a : Inport
  coeff : Inport  Port=2
  ld : Inport  Port=3
  AddSub : xbsIndex_r4/AddSub  mode=Addition or Subtraction  use_carryin=off  use_carryout=off  en=off  latency=0  precision=User Defined  arith_type="Signed  (2's comp)"  n_bits=long_cor_acc_n_bits  bin_pt=0  quantization=Truncate  overflow=Wrap  dbl_ovrd=off  use_behavioral_HDL=on  hw_selection=Fabric  pipelined=off  use_rpm=off  block_type=addsub
      settings: xl_area=[2 0 0 4 0 0 0]  block_version=VER_STRING_GOES_HERE
  Register1 : xbsIndex_r4/Register  init=0  rst=on  en=off  dbl_ovrd=off  block_type=register
      settings: xl_area=[2 4 0 0 0 0 0]  block_version=VER_STRING_GOES_HERE
  Register2 : xbsIndex_r4/Register  init=0  rst=off  en=on  dbl_ovrd=off  block_type=register
      settings: xl_area=[2 4 0 0 0 0 0]  block_version=VER_STRING_GOES_HERE
  bc2 : xbsIndex_r4/Convert  gui_display_data_type=Fixed-point  arith_type=Boolean  n_bits=1  bin_pt=0  float_type=Single  exp_bits=8  fraction_bits=24  quantization=Truncate  overflow=Wrap  en=off  latency=0  dbl_ovrd=off  pipeline=off  block_type=convert
      settings: xl_area=[0 0 0 0 0 0 0]  block_version=VER_STRING_GOES_HERE
  bc3 : xbsIndex_r4/Convert  gui_display_data_type=Fixed-point  arith_type=Boolean  n_bits=1  bin_pt=0  float_type=Single  exp_bits=8  fraction_bits=24  quantization=Truncate  overflow=Wrap  en=off  latency=0  dbl_ovrd=off  pipeline=off  block_type=convert
      settings: xl_area=[0 0 0 0 0 0 0]  block_version=VER_STRING_GOES_HERE
  yn : Outport
  wires:
  a/1 -> bc2/1
  AddSub/1 -> Register1/1, Register2/1
  Register2/1 -> yn/1
  Register1/1 -> AddSub/1
  ld/1 -> bc3/1
  bc3/1 -> Register2/2, Register1/2
  bc2/1 -> AddSub/3
  coeff/1 -> AddSub/2

== system ofdm_txrx_supermimo_coded/OFDM Rx MIMO/Long Corr HP/C1/C15/MAC ==
  a : Inport
  coeff : Inport  Port=2
  ld : Inport  Port=3
  AddSub : xbsIndex_r4/AddSub  mode=Addition or Subtraction  use_carryin=off  use_carryout=off  en=off  latency=0  precision=User Defined  arith_type="Signed  (2's comp)"  n_bits=long_cor_acc_n_bits  bin_pt=0  quantization=Truncate  overflow=Wrap  dbl_ovrd=off  use_behavioral_HDL=on  hw_selection=Fabric  pipelined=off  use_rpm=off  block_type=addsub
      settings: xl_area=[2 0 0 4 0 0 0]  block_version=VER_STRING_GOES_HERE
  Register1 : xbsIndex_r4/Register  init=0  rst=on  en=off  dbl_ovrd=off  block_type=register
      settings: xl_area=[2 4 0 0 0 0 0]  block_version=VER_STRING_GOES_HERE
  Register2 : xbsIndex_r4/Register  init=0  rst=off  en=on  dbl_ovrd=off  block_type=register
      settings: xl_area=[2 4 0 0 0 0 0]  block_version=VER_STRING_GOES_HERE
  bc2 : xbsIndex_r4/Convert  gui_display_data_type=Fixed-point  arith_type=Boolean  n_bits=1  bin_pt=0  float_type=Single  exp_bits=8  fraction_bits=24  quantization=Truncate  overflow=Wrap  en=off  latency=0  dbl_ovrd=off  pipeline=off  block_type=convert
      settings: xl_area=[0 0 0 0 0 0 0]  block_version=VER_STRING_GOES_HERE
  bc3 : xbsIndex_r4/Convert  gui_display_data_type=Fixed-point  arith_type=Boolean  n_bits=1  bin_pt=0  float_type=Single  exp_bits=8  fraction_bits=24  quantization=Truncate  overflow=Wrap  en=off  latency=0  dbl_ovrd=off  pipeline=off  block_type=convert
      settings: xl_area=[0 0 0 0 0 0 0]  block_version=VER_STRING_GOES_HERE
  yn : Outport
  wires:
  a/1 -> bc2/1
  AddSub/1 -> Register1/1, Register2/1
  Register2/1 -> yn/1
  Register1/1 -> AddSub/1
  ld/1 -> bc3/1
  bc3/1 -> Register2/2, Register1/2
  bc2/1 -> AddSub/3
  coeff/1 -> AddSub/2

== system ofdm_txrx_supermimo_coded/OFDM Rx MIMO/Long Corr HP/C1/C16/MAC ==
  a : Inport
  coeff : Inport  Port=2
  ld : Inport  Port=3
  AddSub : xbsIndex_r4/AddSub  mode=Addition or Subtraction  use_carryin=off  use_carryout=off  en=off  latency=0  precision=User Defined  arith_type="Signed  (2's comp)"  n_bits=long_cor_acc_n_bits  bin_pt=0  quantization=Truncate  overflow=Wrap  dbl_ovrd=off  use_behavioral_HDL=on  hw_selection=Fabric  pipelined=off  use_rpm=off  block_type=addsub
      settings: xl_area=[2 0 0 4 0 0 0]  block_version=VER_STRING_GOES_HERE
  Register1 : xbsIndex_r4/Register  init=0  rst=on  en=off  dbl_ovrd=off  block_type=register
      settings: xl_area=[2 4 0 0 0 0 0]  block_version=VER_STRING_GOES_HERE
  Register2 : xbsIndex_r4/Register  init=0  rst=off  en=on  dbl_ovrd=off  block_type=register
      settings: xl_area=[2 4 0 0 0 0 0]  block_version=VER_STRING_GOES_HERE
  bc2 : xbsIndex_r4/Convert  gui_display_data_type=Fixed-point  arith_type=Boolean  n_bits=1  bin_pt=0  float_type=Single  exp_bits=8  fraction_bits=24  quantization=Truncate  overflow=Wrap  en=off  latency=0  dbl_ovrd=off  pipeline=off  block_type=convert
      settings: xl_area=[0 0 0 0 0 0 0]  block_version=VER_STRING_GOES_HERE
  bc3 : xbsIndex_r4/Convert  gui_display_data_type=Fixed-point  arith_type=Boolean  n_bits=1  bin_pt=0  float_type=Single  exp_bits=8  fraction_bits=24  quantization=Truncate  overflow=Wrap  en=off  latency=0  dbl_ovrd=off  pipeline=off  block_type=convert
      settings: xl_area=[0 0 0 0 0 0 0]  block_version=VER_STRING_GOES_HERE
  yn : Outport
  wires:
  a/1 -> bc2/1
  AddSub/1 -> Register1/1, Register2/1
  Register2/1 -> yn/1
  Register1/1 -> AddSub/1
  ld/1 -> bc3/1
  bc3/1 -> Register2/2, Register1/2
  bc2/1 -> AddSub/3
  coeff/1 -> AddSub/2

== system ofdm_txrx_supermimo_coded/OFDM Rx MIMO/Long Corr HP/C1/C2/MAC ==
  a : Inport
  coeff : Inport  Port=2
  ld : Inport  Port=3
  AddSub : xbsIndex_r4/AddSub  mode=Addition or Subtraction  use_carryin=off  use_carryout=off  en=off  latency=0  precision=User Defined  arith_type="Signed  (2's comp)"  n_bits=long_cor_acc_n_bits  bin_pt=0  quantization=Truncate  overflow=Wrap  dbl_ovrd=off  use_behavioral_HDL=on  hw_selection=Fabric  pipelined=off  use_rpm=off  block_type=addsub
      settings: xl_area=[2 0 0 4 0 0 0]  block_version=VER_STRING_GOES_HERE
  Register1 : xbsIndex_r4/Register  init=0  rst=on  en=off  dbl_ovrd=off  block_type=register
      settings: xl_area=[2 4 0 0 0 0 0]  block_version=VER_STRING_GOES_HERE
  Register2 : xbsIndex_r4/Register  init=0  rst=off  en=on  dbl_ovrd=off  block_type=register
      settings: xl_area=[2 4 0 0 0 0 0]  block_version=VER_STRING_GOES_HERE
  bc2 : xbsIndex_r4/Convert  gui_display_data_type=Fixed-point  arith_type=Boolean  n_bits=1  bin_pt=0  float_type=Single  exp_bits=8  fraction_bits=24  quantization=Truncate  overflow=Wrap  en=off  latency=0  dbl_ovrd=off  pipeline=off  block_type=convert
      settings: xl_area=[0 0 0 0 0 0 0]  block_version=VER_STRING_GOES_HERE
  bc3 : xbsIndex_r4/Convert  gui_display_data_type=Fixed-point  arith_type=Boolean  n_bits=1  bin_pt=0  float_type=Single  exp_bits=8  fraction_bits=24  quantization=Truncate  overflow=Wrap  en=off  latency=0  dbl_ovrd=off  pipeline=off  block_type=convert
      settings: xl_area=[0 0 0 0 0 0 0]  block_version=VER_STRING_GOES_HERE
  yn : Outport
  wires:
  a/1 -> bc2/1
  AddSub/1 -> Register1/1, Register2/1
  Register2/1 -> yn/1
  Register1/1 -> AddSub/1
  ld/1 -> bc3/1
  bc3/1 -> Register2/2, Register1/2
  bc2/1 -> AddSub/3
  coeff/1 -> AddSub/2

== system ofdm_txrx_supermimo_coded/OFDM Rx MIMO/Long Corr HP/C1/C3/MAC ==
  a : Inport
  coeff : Inport  Port=2
  ld : Inport  Port=3
  AddSub : xbsIndex_r4/AddSub  mode=Addition or Subtraction  use_carryin=off  use_carryout=off  en=off  latency=0  precision=User Defined  arith_type="Signed  (2's comp)"  n_bits=long_cor_acc_n_bits  bin_pt=0  quantization=Truncate  overflow=Wrap  dbl_ovrd=off  use_behavioral_HDL=on  hw_selection=Fabric  pipelined=off  use_rpm=off  block_type=addsub
      settings: xl_area=[2 0 0 4 0 0 0]  block_version=VER_STRING_GOES_HERE
  Register1 : xbsIndex_r4/Register  init=0  rst=on  en=off  dbl_ovrd=off  block_type=register
      settings: xl_area=[2 4 0 0 0 0 0]  block_version=VER_STRING_GOES_HERE
  Register2 : xbsIndex_r4/Register  init=0  rst=off  en=on  dbl_ovrd=off  block_type=register
      settings: xl_area=[2 4 0 0 0 0 0]  block_version=VER_STRING_GOES_HERE
  bc2 : xbsIndex_r4/Convert  gui_display_data_type=Fixed-point  arith_type=Boolean  n_bits=1  bin_pt=0  float_type=Single  exp_bits=8  fraction_bits=24  quantization=Truncate  overflow=Wrap  en=off  latency=0  dbl_ovrd=off  pipeline=off  block_type=convert
      settings: xl_area=[0 0 0 0 0 0 0]  block_version=VER_STRING_GOES_HERE
  bc3 : xbsIndex_r4/Convert  gui_display_data_type=Fixed-point  arith_type=Boolean  n_bits=1  bin_pt=0  float_type=Single  exp_bits=8  fraction_bits=24  quantization=Truncate  overflow=Wrap  en=off  latency=0  dbl_ovrd=off  pipeline=off  block_type=convert
      settings: xl_area=[0 0 0 0 0 0 0]  block_version=VER_STRING_GOES_HERE
  yn : Outport
  wires:
  a/1 -> bc2/1
  AddSub/1 -> Register1/1, Register2/1
  Register2/1 -> yn/1
  Register1/1 -> AddSub/1
  ld/1 -> bc3/1
  bc3/1 -> Register2/2, Register1/2
  bc2/1 -> AddSub/3
  coeff/1 -> AddSub/2

== system ofdm_txrx_supermimo_coded/OFDM Rx MIMO/Long Corr HP/C1/C4/MAC ==
  a : Inport
  coeff : Inport  Port=2
  ld : Inport  Port=3
  AddSub : xbsIndex_r4/AddSub  mode=Addition or Subtraction  use_carryin=off  use_carryout=off  en=off  latency=0  precision=User Defined  arith_type="Signed  (2's comp)"  n_bits=long_cor_acc_n_bits  bin_pt=0  quantization=Truncate  overflow=Wrap  dbl_ovrd=off  use_behavioral_HDL=on  hw_selection=Fabric  pipelined=off  use_rpm=off  block_type=addsub
      settings: xl_area=[2 0 0 4 0 0 0]  block_version=VER_STRING_GOES_HERE
  Register1 : xbsIndex_r4/Register  init=0  rst=on  en=off  dbl_ovrd=off  block_type=register
      settings: xl_area=[2 4 0 0 0 0 0]  block_version=VER_STRING_GOES_HERE
  Register2 : xbsIndex_r4/Register  init=0  rst=off  en=on  dbl_ovrd=off  block_type=register
      settings: xl_area=[2 4 0 0 0 0 0]  block_version=VER_STRING_GOES_HERE
  bc2 : xbsIndex_r4/Convert  gui_display_data_type=Fixed-point  arith_type=Boolean  n_bits=1  bin_pt=0  float_type=Single  exp_bits=8  fraction_bits=24  quantization=Truncate  overflow=Wrap  en=off  latency=0  dbl_ovrd=off  pipeline=off  block_type=convert
      settings: xl_area=[0 0 0 0 0 0 0]  block_version=VER_STRING_GOES_HERE
  bc3 : xbsIndex_r4/Convert  gui_display_data_type=Fixed-point  arith_type=Boolean  n_bits=1  bin_pt=0  float_type=Single  exp_bits=8  fraction_bits=24  quantization=Truncate  overflow=Wrap  en=off  latency=0  dbl_ovrd=off  pipeline=off  block_type=convert
      settings: xl_area=[0 0 0 0 0 0 0]  block_version=VER_STRING_GOES_HERE
  yn : Outport
  wires:
  a/1 -> bc2/1
  AddSub/1 -> Register1/1, Register2/1
  Register2/1 -> yn/1
  Register1/1 -> AddSub/1
  ld/1 -> bc3/1
  bc3/1 -> Register2/2, Register1/2
  bc2/1 -> AddSub/3
  coeff/1 -> AddSub/2

== system ofdm_txrx_supermimo_coded/OFDM Rx MIMO/Long Corr HP/C1/C5/MAC ==
  a : Inport
  coeff : Inport  Port=2
  ld : Inport  Port=3
  AddSub : xbsIndex_r4/AddSub  mode=Addition or Subtraction  use_carryin=off  use_carryout=off  en=off  latency=0  precision=User Defined  arith_type="Signed  (2's comp)"  n_bits=long_cor_acc_n_bits  bin_pt=0  quantization=Truncate  overflow=Wrap  dbl_ovrd=off  use_behavioral_HDL=on  hw_selection=Fabric  pipelined=off  use_rpm=off  block_type=addsub
      settings: xl_area=[2 0 0 4 0 0 0]  block_version=VER_STRING_GOES_HERE
  Register1 : xbsIndex_r4/Register  init=0  rst=on  en=off  dbl_ovrd=off  block_type=register
      settings: xl_area=[2 4 0 0 0 0 0]  block_version=VER_STRING_GOES_HERE
  Register2 : xbsIndex_r4/Register  init=0  rst=off  en=on  dbl_ovrd=off  block_type=register
      settings: xl_area=[2 4 0 0 0 0 0]  block_version=VER_STRING_GOES_HERE
  bc2 : xbsIndex_r4/Convert  gui_display_data_type=Fixed-point  arith_type=Boolean  n_bits=1  bin_pt=0  float_type=Single  exp_bits=8  fraction_bits=24  quantization=Truncate  overflow=Wrap  en=off  latency=0  dbl_ovrd=off  pipeline=off  block_type=convert
      settings: xl_area=[0 0 0 0 0 0 0]  block_version=VER_STRING_GOES_HERE
  bc3 : xbsIndex_r4/Convert  gui_display_data_type=Fixed-point  arith_type=Boolean  n_bits=1  bin_pt=0  float_type=Single  exp_bits=8  fraction_bits=24  quantization=Truncate  overflow=Wrap  en=off  latency=0  dbl_ovrd=off  pipeline=off  block_type=convert
      settings: xl_area=[0 0 0 0 0 0 0]  block_version=VER_STRING_GOES_HERE
  yn : Outport
  wires:
  a/1 -> bc2/1
  AddSub/1 -> Register1/1, Register2/1
  Register2/1 -> yn/1
  Register1/1 -> AddSub/1
  ld/1 -> bc3/1
  bc3/1 -> Register2/2, Register1/2
  bc2/1 -> AddSub/3
  coeff/1 -> AddSub/2

== system ofdm_txrx_supermimo_coded/OFDM Rx MIMO/Long Corr HP/C1/C6/MAC ==
  a : Inport
  coeff : Inport  Port=2
  ld : Inport  Port=3
  AddSub : xbsIndex_r4/AddSub  mode=Addition or Subtraction  use_carryin=off  use_carryout=off  en=off  latency=0  precision=User Defined  arith_type="Signed  (2's comp)"  n_bits=long_cor_acc_n_bits  bin_pt=0  quantization=Truncate  overflow=Wrap  dbl_ovrd=off  use_behavioral_HDL=on  hw_selection=Fabric  pipelined=off  use_rpm=off  block_type=addsub
      settings: xl_area=[2 0 0 4 0 0 0]  block_version=VER_STRING_GOES_HERE
  Register1 : xbsIndex_r4/Register  init=0  rst=on  en=off  dbl_ovrd=off  block_type=register
      settings: xl_area=[2 4 0 0 0 0 0]  block_version=VER_STRING_GOES_HERE
  Register2 : xbsIndex_r4/Register  init=0  rst=off  en=on  dbl_ovrd=off  block_type=register
      settings: xl_area=[2 4 0 0 0 0 0]  block_version=VER_STRING_GOES_HERE
  bc2 : xbsIndex_r4/Convert  gui_display_data_type=Fixed-point  arith_type=Boolean  n_bits=1  bin_pt=0  float_type=Single  exp_bits=8  fraction_bits=24  quantization=Truncate  overflow=Wrap  en=off  latency=0  dbl_ovrd=off  pipeline=off  block_type=convert
      settings: xl_area=[0 0 0 0 0 0 0]  block_version=VER_STRING_GOES_HERE
  bc3 : xbsIndex_r4/Convert  gui_display_data_type=Fixed-point  arith_type=Boolean  n_bits=1  bin_pt=0  float_type=Single  exp_bits=8  fraction_bits=24  quantization=Truncate  overflow=Wrap  en=off  latency=0  dbl_ovrd=off  pipeline=off  block_type=convert
      settings: xl_area=[0 0 0 0 0 0 0]  block_version=VER_STRING_GOES_HERE
  yn : Outport
  wires:
  a/1 -> bc2/1
  AddSub/1 -> Register1/1, Register2/1
  Register2/1 -> yn/1
  Register1/1 -> AddSub/1
  ld/1 -> bc3/1
  bc3/1 -> Register2/2, Register1/2
  bc2/1 -> AddSub/3
  coeff/1 -> AddSub/2
